simulink model "s_c_nbe_m1k_bu"  (R2016b) (continued)
== system s_c_nbe_m1k_bu == (continued)
  [synced_delay] -> Logical7/2
  [fault_detected] -> Logical7/3
  [sync_timeout] -> Logical14/2
  [auto_rst_enable] -> Logical14/1
  Logical14/1 -> Logical8/2
  Logical7/1 -> Logical8/3
  pipeline23/1(q) -> [outfreq_stopmux]
  ct_debug/6(in_outfreq_stopmux) -> pipeline23/1(d)
  pipeline24/1(q) -> [out_stopmux]
  ct_debug/5(in_out_stopmux) -> pipeline24/1(d)
  pipeline55/1(q) -> [wrrd_stopmux]
  ct_debug/3(in_wrrd_stopmux) -> pipeline55/1(d)
  pipeline56/1(q) -> [in_stopmux]
  ct_debug/1(in_in_stopmux) -> pipeline56/1(d)
  pipeline122/1(q) -> [ct_snap_arm]
  pipeline22/1(q) -> edge_detect7/1
  ct_debug/8(in_snap_trig) -> pipeline22/1(d)
  ct_debug/7(in_snap_arm) -> pipeline122/1(d)
  edge_detect7/1 -> [ct_snap_trig]
  ct_debug/2(in_in_trigmux) -> pipeline150/1(d)
  pipeline150/1(q) -> [in_trigmux]
  ct_debug/4(in_obuf_stopmux) -> pipeline167/1(d)
  pipeline167/1(q) -> [obuf_stopmux]
  ct_debug/9(in_snap_wesel) -> pipeline81/1(d)
  pipeline81/1(q) -> [ct_snap_wesel]
  pipeline157/1(q) -> [ct_snap_trigsel]
  ct_debug/10(in_snap_trigsel) -> pipeline157/1(d)
  pipeline166/1(q) -> [ct_snap_circsel]
  ct_debug/11(in_snap_circsel) -> pipeline166/1(d)
  bus_create2/1(bus_out) -> [tvgtone_level0]
  bus_create3/1(bus_out) -> [tvgtone_level1]
  tvg_tone_levels/2(in_p0i) -> bus_create2/2(in2)
  tvg_tone_levels/3(in_p1r) -> bus_create3/1(in1)
  tvg_tone_levels/4(in_p1i) -> bus_create3/2(in2)
  tvg_tone_levels/1(in_p0r) -> bus_create2/1(in1)
  tvg_tone_targets/1(in_p0) -> Slice18/1
  tvg_tone_targets/2(in_p1) -> Slice19/1
  Constant51/1 -> tvg_tone_targets/1(sim_1)
  Constant52/1 -> tvg_tone_targets/2(sim_2)
  Constant37/1 -> tvg_tone_levels/4(sim_4)
  Constant8/1 -> tvg_tone_levels/1(sim_1)
  Constant27/1 -> tvg_tone_levels/3(sim_3)
  Constant9/1 -> tvg_tone_levels/2(sim_2)
  we_delay49/1(q) -> [ct_tag_insert_sel]
  edge_detect2/1 -> [ct_rst_err_latch]
  we_delay47/1(q) -> edge_detect2/1
  we_delay48/1(q) -> [ct_fake_data_error]
  we_delay13/1(q) -> [ct_tvg_en]
  we_delay50/1(q) -> [ct_tvg_en2]
  Constant12/1 -> ct_control0/5(sim_5)
  Constant21/1 -> ct_control0/4(sim_4)
  Constant20/1 -> ct_control0/3(sim_3)
  Constant22/1 -> ct_control0/2(sim_2)
  Constant15/1 -> ct_control0/1(sim_1)
  ct_control0/1(in_tvg_en) -> we_delay13/1(d)
  ct_control0/2(in_tvg_en2) -> we_delay50/1(d)
  ct_control0/3(in_rst_err_latch) -> we_delay47/1(d)
  ct_control0/4(in_fake_error) -> we_delay48/1(d)
  ct_control0/5(in_tag_insert) -> we_delay49/1(d)
  we_delay126/1(q) -> [obuf_read_gap]
  ct_control0/6(in_obuf_read_gap) -> we_delay126/1(d)
  Constant30/1 -> ct_control0/6(sim_6)
  we_delay41/1(q) -> [ct_num_xeng]
  we_delay112/1(q) -> [ct_num_xeng_recip]  "NUM_X_RECIP"
  ct_control1/2(in_num_x_recip) -> we_delay112/1(d)
  ct_control1/1(in_num_x) -> we_delay41/1(d)
  we_delay114/1(q) -> [ct_x_per_board]
  ct_control1/3(in_x_per_board) -> we_delay114/1(d)
  we_delay113/1(q) -> [ct_chans_per_board]
  ct_control2/1(in_chans_per_x) -> we_delay39/1(d)
  ct_control2/2(in_chans_per_board) -> we_delay113/1(d)
  we_delay115/1(q) -> [ct_x_per_board_recip]
  ct_control1/4(in_x_per_board_recip) -> we_delay115/1(d)
  we_delay38/1(q) -> [ct_num_x_boards]
  ct_control3/1(in_num_x_boards) -> we_delay38/1(d)
  we_delay37/1(q) -> [ct_num_x_boards_recip]
  ct_control3/2(in_num_x_boards_recip) -> we_delay37/1(d)
  ct_control3/3(in_chans_per_x_recip) -> we_delay124/1(d)
  Constant49/1 -> ct_control1/1(sim_1)
  Constant53/1 -> ct_control1/2(sim_2)
  Constant33/1 -> ct_control1/3(sim_3)
  Constant23/1 -> ct_control2/1(sim_1)
  Constant50/1 -> ct_control2/2(sim_2)
  Constant54/1 -> ct_control1/4(sim_4)
  Constant32/1 -> ct_control3/1(sim_1)
  Constant14/1 -> ct_control3/2(sim_2)
  Constant44/1 -> ct_control3/3(sim_3)
  Slice18/1 -> [tvgtone_p0]
  Slice19/1 -> [tvgtone_p1]
  Slice36/1 -> we_delay116/1(d)
  Constant38/1 -> ct_control4/1(sim_1)
  ct_control4/1(in_ct_board_offset) -> Slice36/1
  ct_control5/1(in_ct_freq_gen_offset) -> we_delay117/1(d)
  Constant39/1 -> ct_control5/1(sim_1)
  we_delay116/1(q) -> [ct_board_hmc_offset]
  we_delay117/1(q) -> [ct_freq_gen_offset]
  we_delay124/1(q) -> [ct_chans_per_x_recip]
  we_delay39/1(q) -> [ct_chans_per_x]  "PER_X"
  pipeline196/1(q) -> ddc_dvalid_cnt/1(out_reg)
  pipeline197/1(q) -> ddc_sync_cnt/1(out_reg)
  pipeline198/1(q) -> DDC/8(scale1)
  pipeline199/1(q) -> DDC/5(scale0)
  trig_time_msw/1(in_msw) -> pipeline213/1(d)
  trig_time_lsw/1(in_lsw) -> pipeline214/1(d)
  Concat13/1 -> Relational10/1
  pipeline213/1(q) -> Concat13/1
  pipeline214/1(q) -> Concat13/2
  [time80_48] -> Relational10/2
  pipeline215/1(q) -> bus_expand18/1(bus_in)
  [valid80] -> Slice5/1
  Slice14/1 -> pipeline211/1(d)
  [time80_48] -> Slice14/1
  pipeline211/1(q) -> snap_adc0/13(extra_timestamp)
  [d80_adc0] -> pipeline215/1(d)
  bus_expand18/4(out5) -> snap_adc0/4(in_p0_d3)
  bus_expand18/5(out4) -> snap_adc0/5(in_p0_d4)
  bus_expand18/1(msb_out8) -> Gateway Out3/1, snap_adc0/1(in_p0_d0)
  bus_expand18/2(out7) -> Gateway Out23/1, snap_adc0/2(in_p0_d1)
  bus_expand18/3(out6) -> snap_adc0/3(in_p0_d2)
  bus_expand18/6(out3) -> snap_adc0/6(in_p0_d5)
  bus_expand18/7(out2) -> snap_adc0/7(in_p0_d6)
  bus_expand18/8(lsb_out1) -> snap_adc0/8(in_p0_d7)
  pipeline202/1(q) -> snap_adc0/11(we)
  Relational10/1 -> Logical12/1
  [valid80] -> pipeline162/1(d)
  Logical12/1 -> snap_adc0/12(trig)
  pipeline162/1(q) -> Logical12/2
  [pfbin_sync] -> Delay21/1
  [ddc_sync_in] -> DDC/1(sync)
  [pfbin_d0] -> Delay2/1
  pipeline68/1(q) -> pipeline66/1(d)
  pipeline83/1(q) -> pipeline59/1(d)
  Slice5/1 -> pipeline68/1(d), pipeline202/1(d)
  [sync80] -> pipeline83/1(d)
  [ddc_pol1_in] -> DDC/6(pol1_data)
  Slice17/1 -> local_time_lsw/1(out_timestamp_lsw)
  Slice15/1 -> Convert1/1
  [local_time_capture] -> Delay31/1
  pipeline19/1(q) -> Slice17/1, Slice15/1
  [local_time_src] -> Mux/1
  [time80_48] -> Delay14/1
  [valid80] -> Delay14/2
  Delay14/1 -> Register1/1, AddSub/2
  [pfb_time] -> Delay10/1
  [pfb_dv] -> Delay10/2
  Delay11/1 -> Register3/1, AddSub/1
  [flag_dvalid] -> Delay11/2
  [pack_sync_time] -> Delay11/1
  Convert1/1 -> local_time_msw/1(out_timestamp_msw)
  AddSub/1 -> time_difference/1(out_time_difference), Relational4/1
  Relational4/1 -> Logical/1
  Logical/1 -> [time_diff_error]
  time_check/1(in_max_difference) -> Relational4/2
  [time_diff_check_en] -> Logical/2
  Delay31/1 -> Register3/2, Register2/2, Register4/2, Register1/2
  Delay10/1 -> Register2/1
  [spead_timestamp] -> Register4/1
  Register4/1 -> Mux/2
  Register1/1 -> Mux/3
  Register2/1 -> Mux/4
  Register3/1 -> Mux/5
  Mux/1 -> pipeline19/1(d)
  [cnt_rst] -> Subsystem2/1(rst)
  [synced] -> Delay38/1
  [sync_armed] -> Delay39/1
  [board_in_fault] -> Delay48/1
  [q_sync] -> Subsystem19/5(in3)
  [pfb_sync] -> Subsystem19/3(in1)
  [fdfs_sync] -> Subsystem19/4(in2)
  [sync80] -> Subsystem19/2(in0)
  [cnt_rst] -> Subsystem19/1(rst)
  Subsystem19/2(out1) -> sync_status2/2(out_sync_pfb_cnt)
  Subsystem19/1(out0) -> sync_status2/1(out_sync80_cnt)
  Subsystem19/3(out2) -> sync_status2/3(out_sync_fdfs_cnt)
  [pack_sync] -> Subsystem12/4(in2)
  [flag_sync] -> Subsystem12/3(in1)
  [ct_sync] -> Subsystem12/2(in0)
  [cnt_rst] -> Subsystem12/1(rst)
  [synced_delay] -> Subsystem2/2(armed)
  [sync_timeout] -> Subsystem2/3(in1)
  [time_diff_error] -> Subsystem2/4(in2)
  [up_timestep_error] -> Subsystem2/5(in3)
  [cd_error] -> Subsystem2/6(in4)
  [ct_error] -> Subsystem2/7(in5)
  Delay48/1 -> sync_status0/2(out_board_in_fault)
  Delay38/1 -> sync_status0/3(out_synced)
  Delay39/1 -> sync_status0/4(out_sync_armed)
  Subsystem12/1(out0) -> sync_status0/5(out_sync_ct)
  Subsystem12/2(out1) -> sync_status0/6(out_sync_flag)
  Subsystem12/3(out2) -> sync_status0/7(out_sync_pack)
  Subsystem2/1(Out1) -> sync_status1/1(out_timeout_resync_cnt)
  Subsystem2/2(Out2) -> sync_status1/2(out_time_diff_resync_cnt)
  Subsystem2/3(Out3) -> sync_status1/3(out_timestep_resync_cnt)
  [synced_delay] -> Delay58/1
  Delay58/1 -> sync_status0/1(out_synced_delay)
  Subsystem19/4(out3) -> sync_status2/4(out_sync_quant_cnt)
  Subsystem2/4(Out4) -> sync_status1/4(out_cd_resync_cnt)
  Subsystem2/5(Out5) -> sync_status1/5(out_ct_resync_cnt)
  [clr_status] -> Subsystem3/1(rst)
  Slice6/1 -> Subsystem3/2(in0)
  [pfb_of] -> Slice6/1, Slice20/1
  Slice20/1 -> Subsystem3/3(in1)
  [pfb_sync] -> Subsystem3/4(in2)
  Subsystem3/3(out2) -> pipeline193/1(d)
  Subsystem3/1(out0) -> pipeline195/1(d)
  Subsystem3/2(out1) -> pipeline194/1(d)
  Constant17/1 -> [pfb_of]
  Counter7/1 -> pipeline191/1(d)
  [pfb_sync] -> Counter7/2
  [cnt_rst] -> Counter7/1
  [pfb_dv] -> Counter8/2
  [cnt_rst] -> Counter8/1
  Counter8/1 -> pipeline192/1(d)
  pipeline191/1(q) -> nb_sync_cnt/1(out_reg)
  pipeline192/1(q) -> nb_dvalid_cnt/1(out_reg)
  pipeline193/1(q) -> pfb_status/3(out_sync_cnt)
  pipeline194/1(q) -> pfb_status/2(out_pol1_or_err_cnt)
  pipeline195/1(q) -> pfb_status/1(out_pol0_or_err_cnt)
  Delay72/1 -> [flag_sync]
  Delay75/1 -> [flag_misc]
  Delay73/1 -> [flag_dvalid]
  Delay74/1 -> [flag_time]
  Delay43/1 -> time_gen1/4(rd_sync_in)
  Delay59/1 -> time_gen1/6(rd_misc_in)
  Delay/1 -> Delay43/1  "gapsync"
  Delay3/1 -> Delay47/1  "gapdata"
  Mux1/1 -> Delay59/1  "gapvalid"
  [time80] -> Slice2/1
  Delay47/1 -> time_gen1/5(rd_en)
  time_gen1/1(rd_sync_out) -> Delay72/1
  time_gen1/4(rd_misc_out) -> Delay75/1
  time_gen1/2(rd_dvalid) -> Delay73/1
  time_gen1/3(rd_val_out) -> Delay74/1
  Slice2/1 -> time_gen1/3(wr_val_in)
  [ct_tvg_en2] -> Mux1/1
  Delay17/1 -> Mux1/2
  Delay18/1 -> tvg/1(sync), Delay/1
  Delay16/1 -> tvg/2(dv), Delay3/1
  tvg/1(data256) -> Mux1/3
  [flag_dvalid] -> Subsystem13/1(In1)
  Subsystem13/1(Out1) -> pipeline186/1(d)
  [s256_v] -> Subsystem11/1(In1)
  Subsystem11/1(Out1) -> pipeline187/1(d)
  pipeline38/1(q) -> Convert3/1
  pipeline46/1(q) -> [ip_per_x]
  pipeline47/1(q) -> [num_x]
  x_setup/1(in_f_per_x) -> pipeline38/1(d)
  x_setup/2(in_ip_per_x) -> pipeline46/1(d)
  x_setup/3(in_num_x) -> pipeline47/1(d)
  pipeline52/1(q) -> [pack_snap_arm]
  x_setup/4(in_snap_arm) -> pipeline52/1(d)
  pipeline48/1(q) -> [pktstart_sel]
  x_setup/5(in_pktstart_mux) -> pipeline48/1(d)
  [s256_v] -> pack_block_check/1(dv)
  [cnt_rst] -> Subsystem14/1(rst)
  Subsystem14/1(out0) -> pipeline188/1(d)
  pack_block_check/1(error) -> [pack_dv_err], Subsystem14/2(in0)
  pipeline49/1(q) -> [pack_mux]
  x_setup/6(in_pack_mux) -> pipeline49/1(d)
  Convert3/1 -> [f_per_x]
  [ct_d256] -> Delay17/1
  [ct_dv] -> Delay16/1
  [ct_sync] -> Delay18/1
  x_setup/7(in_pktoffset_sel) -> pipeline54/1(d)
  pipeline54/1(q) -> [pktoffset_sel]
  iptx_base/1(in_reg) -> pipeline53/1(d)
  pipeline53/1(q) -> [tx_ip_base]
  tx_metadata/1(in_porttx) -> pipeline50/1(d)
  tx_metadata/2(in_board_id) -> pipeline51/1(d)
  pipeline50/1(q) -> [tx_port]
  pipeline51/1(q) -> [tx_board_id]
  Delay64/1 -> Delay68/1
  Delay4/1 -> Delay5/1
  Delay5/1 -> Delay60/1
  Delay60/1 -> Delay64/1
  Delay68/1 -> [flag_pktstart]
  Delay1/1 -> Delay6/1
  Delay6/1 -> Delay61/1
  Delay65/1 -> Delay69/1
  Delay69/1 -> [flag_pktfreq]
  Delay61/1 -> Delay65/1
  Delay15/1 -> Delay7/1
  Delay7/1 -> Delay62/1
  Delay62/1 -> Delay66/1
  Delay66/1 -> Delay70/1
  Delay70/1 -> [flag_pktxindex]
  Delay19/1 -> Delay20/1
  Delay20/1 -> Delay63/1
  Delay63/1 -> Delay67/1
  Delay67/1 -> Delay71/1
  Delay71/1 -> [flag_pktxoffset]
  [ct_pkt_x_index] -> Delay15/1
  [ct_pkt_x_offset] -> Delay19/1
  [ct_pktfreq] -> Delay1/1
  [ct_pktstart] -> Delay4/1
  [flag_sync] -> packetiser/1(sync_in)
  [flag_dvalid] -> packetiser/2(en)
  [flag_pktstart] -> packetiser/5(pkt_start)
  [flag_pktxoffset] -> packetiser/7(pkt_x_offset)
  [tx_ip_base] -> packetiser/9(base_ip)
  [tx_port] -> packetiser/10(base_port)
  Constant3/1 -> concat1/2
  [flag_time] -> concat1/1
  [flag_misc] -> packetiser/3(din)
  concat1/1 -> [pack_sync_time], packetiser/4(timestamp)
  Delay82/1 -> [s256_d]
  Delay83/1 -> [s256_v]
  Delay84/1 -> [s256_eof]
  Delay85/1 -> [s256_of]
  Delay86/1 -> [s256_ip]
  Delay87/1 -> [s256_port]
  Delay88/1 -> [pack_sync]
  packetiser/1(dout) -> Delay82/1
  packetiser/2(dvalid) -> Delay83/1
  packetiser/3(eof) -> Delay84/1
  packetiser/4(overflow) -> Delay85/1
  packetiser/5(destination_ip) -> Delay86/1
  packetiser/6(destination_port) -> Delay87/1
  packetiser/7(sync) -> Delay88/1
  [flag_pktfreq] -> Delay77/1
  Delay89/1 -> [s256_pktfreq]
  Delay77/1 -> Delay89/1
  [flag_pktxindex] -> Delay78/1
  Delay78/1 -> Delay90/1
  Delay90/1 -> [s256_pktxindex]
  [flag_pktxoffset] -> Delay79/1
  Delay79/1 -> Delay91/1
  Delay91/1 -> [s256_pktxoffset]
  [tx_board_id] -> packetiser/8(board_id)
  [flag_pktstart] -> Delay80/1
  Delay80/1 -> Delay92/1
  Delay92/1 -> [s256_pktstart]
  [sync80] -> time_gen1/1(wr_sync_in)
  [valid80] -> time_gen1/2(wr_en)
  [time80_48] -> Subsystem/1(time)
  [sync80] -> Subsystem/2(sync)
  [valid80] -> Subsystem/3(dv)
  Subsystem/1(msw) -> pipeline184/1(d)
  Subsystem/2(ls32) -> pipeline185/1(d)
  [flag_pktxindex] -> packetiser/6(pkt_x_index)
  [flag_time] -> Delay81/1
  Delay81/1 -> Delay93/1
  Delay93/1 -> [s256_time]
  time_gen1/5(epoch) -> Delay76/1
  Slice4/1 -> pipeline182/1(d)
  Slice11/1 -> pipeline183/1(d)
  Delay76/1 -> Concat10/1
  Constant16/1 -> Concat10/2
  Concat10/1 -> Slice4/1, Slice11/1, [flag_epoch]
  [flag_pktfreq] -> Subsystem27/3(value)
  [cnt_rst] -> Subsystem27/1(rst)
  [flag_dvalid] -> Subsystem27/2(en)
  Subsystem27/1(current) -> pipeline170/1(d)
  Subsystem27/3(min) -> pipeline172/1(d)
  Subsystem27/2(max) -> pipeline171/1(d)
  [flag_pktxindex] -> Subsystem28/3(value)
  [cnt_rst] -> Subsystem28/1(rst)
  [flag_dvalid] -> Subsystem28/2(en)
  Subsystem28/1(current) -> pipeline173/1(d)
  Subsystem28/3(min) -> pipeline175/1(d)
  Subsystem28/2(max) -> pipeline174/1(d)
  [flag_pktxoffset] -> Subsystem29/3(value)
  [cnt_rst] -> Subsystem29/1(rst)
  [flag_dvalid] -> Subsystem29/2(en)
  Subsystem29/1(current) -> pipeline176/1(d)
  Subsystem29/3(min) -> pipeline178/1(d)
  Subsystem29/2(max) -> pipeline177/1(d)
  [flag_time] -> Subsystem30/3(value)
  [cnt_rst] -> Subsystem30/1(rst)
  [flag_pktstart] -> Subsystem30/2(en)
  Subsystem30/1(current) -> pipeline179/1(d)
  Subsystem30/3(min) -> pipeline181/1(d)
  Subsystem30/2(max) -> pipeline180/1(d)
  pipeline170/1(q) -> flag_pktfreq_stats/1(out_current)
  pipeline171/1(q) -> flag_pktfreq_stats/2(out_max)
  pipeline172/1(q) -> flag_pktfreq_stats/3(out_min)
  pipeline175/1(q) -> flag_pktxindex_stats/3(out_min)
  pipeline174/1(q) -> flag_pktxindex_stats/2(out_max)
  pipeline173/1(q) -> flag_pktxindex_stats/1(out_current)
  pipeline176/1(q) -> flag_pktxoffset_stats/1(out_current)
  pipeline177/1(q) -> flag_pktxoffset_stats/2(out_max)
  pipeline178/1(q) -> flag_pktxoffset_stats/3(out_min)
  pipeline181/1(q) -> flag_time_stats/3(out_min)
  pipeline180/1(q) -> flag_time_stats/2(out_max)
  pipeline179/1(q) -> flag_time_stats/1(out_current)
  pipeline182/1(q) -> flag_epoch_lsw/1(out_reg)
  pipeline183/1(q) -> flag_epoch_msw/1(out_reg)
  pipeline184/1(q) -> sync_time_msw/1(out_reg)
  pipeline185/1(q) -> sync_time_lsw/1(out_reg)
  pipeline186/1(q) -> pack_in_dv_rate/1(out_dv_rate)
  pipeline187/1(q) -> pack_out_dv_rate/1(out_dv_rate)
  pipeline188/1(q) -> pack_dv_err/1(out_dvblock_err_cnt)
  [pfbin_sync] -> Logical9/2
  [sys_rst] -> Logical9/1
  Logical9/1 -> pipeline147/1(d)
  [osc_cos_ddc0] -> DDC/7(osc1)
  Mux2/1 -> DDC/4(osc0)
  [osc_cos_ddc0] -> Mux2/2
  [ddc_pol0_in] -> Mux2/3
  Constant11/1 -> osc_sel/1(sim_1)
  osc_sel/1(in_sel) -> Mux2/1
  [pfbin_dv] -> Delay9/1
  Delay2/1 -> [ddc_pol0_in]
  Delay9/1 -> [ddc_dv_in]
  Delay21/1 -> [ddc_sync_in]
  [ddc_pol0] -> nb_pfb/2(pol0)
  [ddc_pol1] -> nb_pfb/3(pol1)
  nb_pfb/2(pol0_out) -> [pfb_d144_0]
  nb_pfb/3(pol1_out) -> [pfb_d144_1]
  [ddc_sync] -> nb_pfb/1(sync)
  nb_pfb/1(sync_out) -> [pfb_sync]
  [ddc_dvalid] -> nb_pfb/4(dvalid)
  nb_pfb/4(dv_out) -> [pfb_dv]
  pipeline204/1(q) -> snap_fft/5(we)
  pipeline203/1(q) -> snap_fft/1(in_real)
  pipeline206/1(q) -> snap_fft/2(in_imag)
  pipeline20/1(q) -> snap_fft/3(in_sync)
  pipeline216/1(q) -> snap_fft/4(in_dv)
  bus_expand1/2(lsb_out1) -> pipeline206/1(d)
  bus_expand1/1(msb_out2) -> pipeline203/1(d)
  bus_expand2/1(msb_out8) -> pipeline142/1(d)
  bus_expand2/2(out7) -> pipeline144/1(d)
  bus_expand2/3(out6) -> pipeline145/1(d)
  bus_expand2/4(out5) -> pipeline146/1(d)
  bus_expand2/5(out4) -> pipeline151/1(d)
  bus_expand2/6(out3) -> pipeline152/1(d)
  pipeline142/1(q) -> snap_nb/1(in_real0)
  pipeline144/1(q) -> snap_nb/2(in_imag0)
  pipeline146/1(q) -> snap_nb/4(in_imag1)
  pipeline151/1(q) -> snap_nb/5(in_real2)
  pipeline152/1(q) -> snap_nb/6(in_imag2)
  pipeline4/1(q) -> snap_nb/7(we)
  generate_pkt_sync1/2(out_valid) -> pipeline34/1(d)
  pipeline154/1(q) -> snap_nb1/1(in_real3)
  pipeline217/1(q) -> snap_nb1/2(in_imag3)
  bus_expand2/7(out2) -> pipeline154/1(d)
  bus_expand2/8(lsb_out1) -> pipeline217/1(d)
  pipeline5/1(q) -> snap_nb1/5(we)
  pipeline229/1(q) -> snap_nb1/4(in_dv)
  pipeline230/1(q) -> snap_nb1/3(in_sync)
  generate_pkt_sync1/4(packet_sync) -> pipeline36/1(d)
  [ddc_dv_in] -> DDC/2(dvalid)
  pipeline145/1(q) -> snap_nb/3(in_real1)
  [ddc_pol0_in] -> DDC/3(pol0_data)
  [pfbin_d1] -> Delay22/1
  [pfbin_dv] -> cwg1/6(en)
  Delay22/1 -> [ddc_pol1_in]
  pipeline106/1(q) -> [osc_temp]
  cwg2/1(bus_out) -> pipeline106/1(d)
  cwg2/3(rpt_rst) -> Terminator15/1
  cwg2/2(dvalid) -> Terminator8/1
  Constant56/1 -> freq_cwg_osc1/1(sim_1)
  Constant58/1 -> scale_cwg_osc1/1(sim_1)
  freq_cwg_osc1/1(in_frequency) -> pipeline121/1(d)
  scale_cwg_osc1/1(in_scale) -> pipeline99/1(d)
  pipeline119/1(q) -> cwg2/3(rst)
  rpt_en_cwg_osc1/1(in_en) -> pipeline123/1(d)
  rpt_length_cwg_osc1/1(in_repeat_length) -> pipeline105/1(d)
  Constant59/1 -> rpt_en_cwg_osc1/1(sim_1)
  Constant60/1 -> rpt_length_cwg_osc1/1(sim_1)
  pipeline99/1(q) -> cwg2/2(scale)
  pipeline121/1(q) -> cwg2/1(freq)
  pipeline123/1(q) -> cwg2/4(repeat_en)
  pipeline105/1(q) -> cwg2/5(repeat_length)
  [pfbin_sync] -> Logical10/2
  [sys_rst] -> Logical10/1
  Logical10/1 -> pipeline119/1(d)
  Gateway Out3/1 -> To Workspace4/1
  Gateway Out23/1 -> To Workspace23/1
  bus_expand6/4(out5) -> pipeline139/1(d)
  bus_expand6/5(out4) -> pipeline133/1(d)
  bus_expand6/2(out7) -> pipeline141/1(d)
  bus_expand6/3(out6) -> pipeline140/1(d)
  bus_expand6/6(out3) -> pipeline130/1(d)
  bus_expand6/7(out2) -> pipeline129/1(d)
  bus_expand6/8(lsb_out1) -> pipeline126/1(d)
  bus_expand6/1(msb_out8) -> pipeline149/1(d)
  pipeline153/1(q) -> snap_hmc_in/12(we)
  pipeline124/1(q) -> snap_hmc_in/10(in_dv)
  pipeline125/1(q) -> snap_hmc_in/9(in_sync)
  pipeline126/1(q) -> snap_hmc_in/8(in_p0_d7)
  pipeline129/1(q) -> snap_hmc_in/7(in_p0_d6)
  pipeline130/1(q) -> snap_hmc_in/6(in_p0_d5)
  pipeline133/1(q) -> snap_hmc_in/5(in_p0_d4)
  pipeline139/1(q) -> snap_hmc_in/4(in_p0_d3)
  pipeline140/1(q) -> snap_hmc_in/3(in_p0_d2)
  pipeline141/1(q) -> snap_hmc_in/2(in_p0_d1)
  pipeline149/1(q) -> snap_hmc_in/1(in_p0_d0)
  From131/1 -> pipeline125/1(d), snap_hmc_in/13(trig)
  From129/1 -> pipeline124/1(d), pipeline153/1(d)
  pipeline161/1(q) -> snap_hmc_in/11(in_en)
  From189/1 -> pipeline161/1(d)
  From186/1 -> bus_expand6/1(bus_in)
  Mux7/1 -> Delay36/1, cwg2/6(en)
  Delay36/1 -> Gateway Out28/1, [osc_temp_dv]
  Counter10/1 -> Relational5/1, Relational2/1, Gateway Out26/1
  Constant62/1 -> osc_temp_dv_sel/1(sim_1)
  osc_temp_dv_sel/1(in_sel) -> Mux7/1
  Constant/1 -> Mux7/2
  Relational2/1 -> Mux7/3, Gateway Out29/1
  Gateway Out28/1 -> To Workspace28/1
  Register5/1 -> edge_detect10/1(in)
  [osc_temp_dv] -> Register5/3, Register5/1
  [sys_rst] -> Register5/2
  Mux8/1 -> [sync80]
  edge_detect10/1(out) -> Mux8/3
  Mux9/1 -> [valid80]
  [osc_temp_dv] -> Delay24/1
  Mux10/1 -> [d80_adc0]
  [osc_temp] -> Delay25/1
  Constant61/1 -> dbg_sel/1(sim_1)
  dbg_sel/1(in_sel) -> Mux8/1, Mux9/1, Mux10/1
  From77/1 -> bus_expand1/1(bus_in)
  From46/1 -> pipeline204/1(d), pipeline216/1(d)
  From45/1 -> snap_fft/6(trig), pipeline20/1(d)
  Counter11/1 -> pipeline233/1(d)
  From98/1 -> Counter11/2
  From117/1 -> Counter11/1
  From101/1 -> Counter13/2
  From118/1 -> Counter13/1
  bus_expand9/4(out5) -> pipeline241/1(d)
  bus_expand9/5(out4) -> pipeline240/1(d)
  bus_expand9/2(out7) -> pipeline243/1(d)
  bus_expand9/3(out6) -> pipeline242/1(d)
  bus_expand9/6(out3) -> pipeline239/1(d)
  bus_expand9/7(out2) -> pipeline238/1(d)
  bus_expand9/8(lsb_out1) -> pipeline32/1(d)
  bus_expand9/1(msb_out8) -> pipeline244/1(d)
  pipeline245/1(q) -> snap_hmc_out/12(we)
  pipeline21/1(q) -> snap_hmc_out/10(in_dv)
  pipeline26/1(q) -> snap_hmc_out/9(in_sync)
  pipeline32/1(q) -> snap_hmc_out/8(in_p0_d7)
  pipeline238/1(q) -> snap_hmc_out/7(in_p0_d6)
  pipeline239/1(q) -> snap_hmc_out/6(in_p0_d5)
  pipeline240/1(q) -> snap_hmc_out/5(in_p0_d4)
  pipeline241/1(q) -> snap_hmc_out/4(in_p0_d3)
  pipeline242/1(q) -> snap_hmc_out/3(in_p0_d2)
  pipeline243/1(q) -> snap_hmc_out/2(in_p0_d1)
  pipeline244/1(q) -> snap_hmc_out/1(in_p0_d0)
  From241/1 -> pipeline246/1(d)
  pipeline246/1(q) -> snap_hmc_out/11(in_err)
  From235/1 -> bus_expand9/1(bus_in)
  From232/1 -> pipeline245/1(d), pipeline21/1(d)
  From239/1 -> snap_hmc_out/13(trig), pipeline26/1(d)
  Counter15/1 -> pipeline236/1(d)
  [ddc_sync_in] -> Counter15/2
  [sys_rst] -> Counter15/1
  tl_fd0/2(enable) -> phase_compensation0/5(load_value)
  tl_fd1/1(value) -> phase_compensation1/4(delay_value)
  tl_fd1/2(enable) -> phase_compensation1/5(load_value)
  tl_fd0/1(value) -> phase_compensation0/4(delay_value)
  [clr_status] -> tl_fd1/1(rst), tl_fd0/1(rst)
  phase_compensation0/1(sync_out) -> [fdfs_sync]
  phase_compensation1/1(sync_out) -> Terminator24/1
  phase_compensation0/2(dout) -> [fdfs_d144_0]
  phase_compensation1/2(dout) -> [fdfs_d144_1]
  phase_compensation0/3(dvalid) -> [fdfs_dv]
  phase_compensation1/3(dvalid) -> Terminator25/1
  [ld_val_fd0] -> tl_fd0/3(load_value)
  [ld_val_fd1] -> tl_fd1/3(load_value)
  bus_expand16/1(msb_out2) -> tl_fd0/5(din)
  tl_fd0/5(dvalid) -> phase_compensation0/3(en)
  tl_fd0/3(sync_out) -> phase_compensation0/1(sync_in)
  tl_fd0/4(dout) -> phase_compensation0/2(din)
  tl_fd1/4(dout) -> phase_compensation1/2(din)
  bus_expand16/2(lsb_out1) -> tl_fd1/5(din)
  Constant66/1 -> Concat2/2
  Concat2/1 -> tl_fd1/2(current_time), tl_fd0/2(current_time), [pfb_time]
  Counter2/1 -> pipeline41/1(d)
  Constant65/1 -> eq1/2(data_in)
  Constant24/1 -> eq1/3(we)
  Delay53/1 -> quant1/1(sync_in)
  Delay37/1 -> quant1/2(din)
  eq1/1(data_out) -> quant1/4(coeffs)
  Delay50/1 -> quant1/3(en)
  Counter4/1 -> pipeline29/1(d)
  Constant63/1 -> eq0/2(data_in)
  Constant64/1 -> eq0/3(we)
  Delay51/1 -> quant0/1(sync_in)
  Delay35/1 -> quant0/2(din)
  Delay52/1 -> quant0/3(en)
  quant0/1(sync_out) -> [q_sync]
  quant0/2(dout) -> [q_d64_0]
  quant0/3(dvalid) -> [q_dv]
  quant0/4(of) -> [q_of0]
  quant1/2(dout) -> [q_d64_1]
  quant1/1(sync_out) -> Terminator19/1
  quant1/3(dvalid) -> Terminator21/1
  quant1/4(of) -> [q_of1]
  pipeline29/1(q) -> eq0/1(addr)
  pipeline41/1(q) -> eq1/1(addr)
  [fdfs_sync] -> Delay51/1, pipeline45/1(d)
  [fdfs_d144_0] -> Delay35/1
  [fdfs_dv] -> Delay52/1, pipeline42/1(d)
  pipeline42/1(q) -> Counter4/2
  pipeline45/1(q) -> Counter4/1
  pipeline189/1(q) -> Counter2/2
  [fdfs_sync] -> Delay53/1, pipeline200/1(d)
  [fdfs_d144_1] -> Delay37/1
  [fdfs_dv] -> Delay50/1, pipeline189/1(d)
  pipeline200/1(q) -> Counter2/1
  [clr_status] -> Subsystem15/1(rst)
  tl_fd0/7(misco) -> Terminator3/1
  tl_fd0/6(time) -> Terminator4/1
  tl_fd1/6(time) -> Terminator17/1
  tl_fd1/3(sync_out) -> phase_compensation1/1(sync_in)
  tl_fd1/5(dvalid) -> phase_compensation1/3(en)
  tl_fd1/7(misco) -> Terminator20/1
  Constant26/1 -> tl_fd0/7(misci)
  Constant29/1 -> tl_fd1/7(misci)
  time_gen/1(rd_sync_out) -> tl_fd1/4(sync_in), tl_fd0/4(sync_in)
  time_gen/2(rd_dvalid) -> tl_fd1/6(en), tl_fd0/6(en)
  time_gen/3(rd_val_out) -> Concat2/1
  time_gen/4(rd_misc_out) -> bus_expand16/1(bus_in)
  [sync80] -> pipeline207/1(d)
  [valid80] -> pipeline208/1(d)
  [pfb_sync] -> pipeline210/1(d)
  [pfb_dv] -> pipeline212/1(d)
  [pfb_d144_1] -> pipeline231/1(d)
  [pfb_d144_0] -> pipeline228/1(d)
  Concat1/1 -> time_gen/6(rd_misc_in)
  [time80] -> pipeline209/1(d)
  Subsystem15/1(out0) -> quant_status/1(out_pol0_or_cnt)
  Relational/1 -> Subsystem15/2(in0)
  Constant4/1 -> Relational/2
  [q_of0] -> Relational/1
  Relational3/1 -> Subsystem15/3(in1)
  [q_of1] -> Relational3/1
  Constant25/1 -> Relational3/2
  Subsystem15/2(out1) -> quant_status/2(out_pol1_or_cnt)
  [q_sync] -> Gateway Out36/1, generate_pkt_sync0/1(sync)
  Concat/1 -> generate_pkt_sync0/3(data)
  generate_pkt_sync0/1(out_sync) -> Terminator18/1
  [q_d64_0] -> Concat/1
  [q_d64_1] -> Concat/2
  bus_expand14/1(msb_out2) -> bus_expand12/1(bus_in)
  [q_dv] -> Gateway Out37/1, generate_pkt_sync0/2(valid)
  generate_pkt_sync0/3(out_data) -> pipeline190/1(d)
  generate_pkt_sync0/2(out_valid) -> pipeline201/1(d)
  generate_pkt_sync0/4(packet_sync) -> pipeline205/1(d)
  pipeline190/1(q) -> bus_expand14/1(bus_in)
  pipeline201/1(q) -> Gateway Out31/1, snap_quant0/9(we)
  pipeline205/1(q) -> Gateway Out27/1, snap_quant0/10(trig)
  bus_expand12/1(msb_out8) -> Gateway Out32/1, snap_quant0/1(in_real0)
  bus_expand12/2(out7) -> Gateway Out33/1, snap_quant0/2(in_imag0)
  bus_expand12/3(out6) -> snap_quant0/3(in_real1)
  bus_expand12/4(out5) -> snap_quant0/4(in_imag1)
  bus_expand12/5(out4) -> snap_quant0/5(in_real2)
  bus_expand12/6(out3) -> snap_quant0/6(in_imag2)
  bus_expand12/7(out2) -> Gateway Out34/1, snap_quant0/7(in_real3)
  bus_expand12/8(lsb_out1) -> Gateway Out35/1, snap_quant0/8(in_imag3)
  bus_expand13/1(msb_out8) -> snap_quant1/1(in_real0)
  bus_expand13/2(out7) -> snap_quant1/2(in_imag0)
  bus_expand13/3(out6) -> snap_quant1/3(in_real1)
  bus_expand13/4(out5) -> snap_quant1/4(in_imag1)
  bus_expand13/5(out4) -> snap_quant1/5(in_real2)
  bus_expand13/6(out3) -> snap_quant1/6(in_imag2)
  bus_expand13/7(out2) -> snap_quant1/7(in_real3)
  bus_expand13/8(lsb_out1) -> snap_quant1/8(in_imag3)
  [tl_cd0_arm] -> tl_fd0/8(arm)
  [tl_cd0_load_immediate] -> tl_fd0/9(load_immediate)
  [tl_cd0_load_time] -> tl_fd0/10(load_time)
  [tl_cd1_arm] -> tl_fd1/8(arm)
  [tl_cd1_load_immediate] -> tl_fd1/9(load_immediate)
  [tl_cd1_load_time] -> tl_fd1/10(load_time)
  pipeline207/1(q) -> time_gen/1(wr_sync_in)
  pipeline208/1(q) -> time_gen/2(wr_en)
  pipeline209/1(q) -> time_gen/3(wr_val_in)
  pipeline210/1(q) -> time_gen/4(rd_sync_in)
  pipeline212/1(q) -> time_gen/5(rd_en)
  pipeline228/1(q) -> Concat1/1
  pipeline231/1(q) -> Concat1/2
  Counter13/1 -> pipeline232/1(d)
  pipeline233/1(q) -> hmc_out_sync_cnt/1(out_reg)
  pipeline236/1(q) -> ddc_in_sync_cnt/1(out_reg)
  pipeline232/1(q) -> sync80_cnt/1(out_reg)
  Counter14/1 -> pipeline237/1(d)
  [q_sync] -> Counter14/2
  [sys_rst] -> Counter14/1
  pipeline237/1(q) -> quant_sync_cnt/1(out_reg)
  From_pfb_dv1/1 -> bus_expand13/1(bus_in)
  From_pfb_dv2/1 -> snap_quant1/9(we)
  From_pfb_sync2/1 -> snap_quant1/10(trig)
  Concat4/1 -> [ld_val_fd0]
  Concat9/1 -> [ld_val_fd1]
  fd0_phase/1(in_initial) -> Reinterpret10/1
  fd0_delta_delay/1(in_delta) -> Reinterpret9/1
  fd0_phase/2(in_delta) -> Reinterpret11/1
  Reinterpret16/1 -> Concat4/1
  Reinterpret9/1 -> Concat4/2
  Reinterpret10/1 -> Concat4/3
  Reinterpret11/1 -> Concat4/4
  fd1_phase/1(in_initial) -> Reinterpret13/1
  fd1_delta_delay/1(in_delta) -> Reinterpret12/1
  fd1_phase/2(in_delta) -> Reinterpret14/1
  Reinterpret12/1 -> Concat9/2
  Reinterpret13/1 -> Concat9/3
  Reinterpret14/1 -> Concat9/4
  Reinterpret15/1 -> Concat9/1
  fd0_delay/1(in_initial) -> Reinterpret16/1
  fd1_delay/1(in_initial) -> Reinterpret15/1
  [ct_dv] -> Subsystem21/1(In1)
  Subsystem21/1(Out1) -> ct_out_dv_rate1/1(out_reg)
  [ct_pktstart] -> we_delay3/1(d)
  [ct_dv] -> we_delay4/1(d)
  we_delay3/1(q) -> pktlen_check1/1(pkt_start)
  we_delay4/1(q) -> dv_block_check1/1(dv), pktlen_check1/2(dv)
  pktlen_check1/1(error) -> we_delay6/1(d)
  dv_block_check1/1(error) -> we_delay5/1(d)
  [cnt_rst] -> Subsystem20/1(rst)
  we_delay6/1(q) -> [ct_pktlen_err], Subsystem20/2(in0)
  we_delay5/1(q) -> [ct_dvblk_err], Subsystem20/3(in1)
  Subsystem20/1(out0) -> ct_dv_err1/1(out_pktlen_err_cnt)
  Subsystem20/2(out1) -> ct_dv_err1/2(out_dvblock_err_cnt)
  [q_dv] -> Subsystem18/1(In1)
  Subsystem18/1(Out1) -> ct_in_dv_rate1/1(out_reg)
  [q_sync] -> hmc_ct/1(in_sync)
  [q_dv] -> hmc_ct/2(in_dv)
  [q_d64_0] -> hmc_ct/3(in_d0)
  [q_d64_1] -> hmc_ct/4(in_d1)
  hmc_ct/1(out_sync) -> [ct_sync]
  hmc_ct/2(out_dv) -> [ct_dv]
  hmc_ct/3(out_d256) -> [ct_d256]
  hmc_ct/4(pkt_start) -> [ct_pktstart]
  hmc_ct/5(freq_id) -> [ct_pktfreq]
  hmc_ct/6(x_index) -> [ct_pkt_x_index]
  hmc_ct/7(x_pkt_index) -> [ct_pkt_x_offset]
  hmc_ct/8(error0) -> [ct_error]
  [cnt_rst] -> Subsystem16/1(rst)
  [q_sync] -> Subsystem16/2(in0)
  Constant67/1 -> Subsystem16/3(in1)
  Constant68/1 -> Subsystem16/4(in2)
  [ct_sync] -> Subsystem16/5(in3)
  Subsystem16/1(out0) -> ct_sync_cnt1/1(out_sync_in)
  Subsystem16/2(out1) -> ct_sync_cnt1/2(out_rd_addr_arm_cnt)
  Subsystem16/3(out2) -> ct_sync_cnt1/3(out_rd_sync_cnt)
  Subsystem16/4(out3) -> ct_sync_cnt1/4(out_sync_out)
  munge_sel/1(in_sel) -> pipeline25/1(d)
  pipeline25/1(q) -> [munge_sel]
  Convert2/1 -> [cd_bypass]
  Delay24/1 -> Mux9/3
  Delay25/1 -> Mux10/3
  [pfb_sync] -> generate_pkt_sync1/1(sync)
  [pfb_dv] -> generate_pkt_sync1/2(valid)
  [pfb_d144_0] -> generate_pkt_sync1/3(data)
  generate_pkt_sync1/3(out_data) -> pipeline35/1(d)
  generate_pkt_sync1/1(out_sync) -> Terminator2/1
  pipeline16/1(q) -> snap_fft1/7(we)
  pipeline11/1(q) -> snap_fft1/1(in_real)
  pipeline27/1(q) -> snap_fft1/2(in_imag)
  pipeline10/1(q) -> snap_fft1/5(in_sync)
  pipeline33/1(q) -> snap_fft1/6(in_dv)
  bus_expand3/2(lsb_out1) -> pipeline27/1(d)
  bus_expand3/1(msb_out2) -> pipeline11/1(d)
  generate_pkt_sync2/3(out_data) -> pipeline40/1(d)
  generate_pkt_sync2/2(out_valid) -> pipeline37/1(d)
  generate_pkt_sync2/4(packet_sync) -> pipeline44/1(d)
  [data_pol0] -> generate_pkt_sync2/3(data)
  [fft_sync] -> generate_pkt_sync2/1(sync)
  [fft_dvalid] -> generate_pkt_sync2/2(valid)
  generate_pkt_sync2/1(out_sync) -> Terminator5/1
  pipeline34/1(q) -> pipeline4/1(d), Gateway Out38/1, pipeline5/1(d), pipeline229/1(d)
  pipeline35/1(q) -> bus_expand2/1(bus_in)
  pipeline36/1(q) -> pipeline230/1(d), Gateway Out39/1, snap_nb1/6(trig), snap_nb/8(trig)
  pipeline37/1(q) -> pipeline33/1(d), pipeline16/1(d)
  pipeline40/1(q) -> bus_expand3/1(bus_in)
  pipeline44/1(q) -> pipeline10/1(d), snap_fft1/8(trig)
  [sync80] -> [pfbin_sync]
  [valid80] -> [pfbin_dv]
  [d80_adc0] -> [pfbin_d0]
  [d80_adc1] -> [pfbin_d1]
  Constant5/1 -> [cd_error]
  Constant10/1 -> munge_sel/1(sim_1)
  pipeline59/1(q) -> snap_adc0/9(in_sync)
  pipeline66/1(q) -> snap_adc0/10(in_dv)
  pipeline8/1(q) -> [unpack_dv]
  pipeline17/1(q) -> [unpack_timestamp]
  pipeline14/1(q) -> [tvg_adc0_en]
  impulse_gen0/1(sync_out) -> Delay23/1, Mux3/2
  Mux3/1 -> Mux8/2
  Delay23/1 -> Mux3/3
  Mux4/1 -> Mux9/2
  impulse_gen0/3(dvalid) -> Delay26/1, Mux4/2
  Delay26/1 -> Mux4/3
  Constant13/1 -> dv_del_sel/1(sim_1)
  dv_del_sel/1(in_sel) -> Mux4/1
  Constant18/1 -> sync_del_sel/1(sim_1)
  sync_del_sel/1(in_sel) -> Mux3/1
  Mux5/1 -> Mux10/2
  Delay27/1 -> Mux5/3
  Constant19/1 -> d0_del_sel/1(sim_1)
  d0_del_sel/1(in_sel) -> Mux5/1
  pipeline72/1(q) -> bus_expand4/1(bus_in)
  From8/1 -> Slice3/1
  Slice1/1 -> pipeline71/1(d)
  From33/1 -> Slice1/1
  pipeline71/1(q) -> snap_adc1/13(extra_timestamp)
  From35/1 -> pipeline72/1(d)
  bus_expand4/4(out5) -> snap_adc1/4(in_p0_d3)
  bus_expand4/5(out4) -> snap_adc1/5(in_p0_d4)
  bus_expand4/1(msb_out8) -> snap_adc1/1(in_p0_d0)
  bus_expand4/2(out7) -> snap_adc1/2(in_p0_d1)
  bus_expand4/3(out6) -> snap_adc1/3(in_p0_d2)
  bus_expand4/6(out3) -> snap_adc1/6(in_p0_d5)
  bus_expand4/7(out2) -> snap_adc1/7(in_p0_d6)
  bus_expand4/8(lsb_out1) -> snap_adc1/8(in_p0_d7)
  pipeline67/1(q) -> snap_adc1/11(we)
  pipeline77/1(q) -> pipeline76/1(d)
  pipeline79/1(q) -> snap_adc1/12(trig), pipeline75/1(d)
  Slice3/1 -> pipeline67/1(d), pipeline77/1(d)
  From34/1 -> pipeline79/1(d)
  pipeline75/1(q) -> snap_adc1/9(in_sync)
  pipeline76/1(q) -> snap_adc1/10(in_dv)
  Mux6/1 -> [osc_cos_ddc0]
  pipeline13/1(q) -> munge/1(din), Mux6/2
  munge/1(dout) -> Mux6/3
  Constant28/1 -> osc_munge/1(sim_1)
  osc_munge/1(in_sel) -> Mux6/1
  impulse_gen0/2(dout) -> Mux11/2, munge1/1(din)
  munge1/1(dout) -> Mux11/3
  Constant31/1 -> adc_munge/1(sim_1)
  adc_munge/1(in_sel) -> Mux11/1
  Mux11/1 -> Delay27/1, Mux5/2
  [ct_d256] -> bus_expand8/1(bus_in)
  [ct_pktfreq] -> pipeline80/1(d)
  [ct_pkt_x_index] -> pipeline84/1(d)
  [ct_freq_sel_dv] -> pipeline87/1(d)
  [ct_pktstart] -> pipeline82/1(d)
  [ct_pkt_x_offset] -> pipeline85/1(d)
  [ct_error] -> pipeline88/1(d)
  [ct_sync] -> pipeline86/1(d)
  pipeline80/1(q) -> snap_ct/1(in_pkt_freq)
  pipeline82/1(q) -> snap_ct/2(in_pkt_start)
  pipeline84/1(q) -> snap_ct/3(in_x_idx)
  pipeline85/1(q) -> snap_ct/4(in_x_offset)
  pipeline86/1(q) -> snap_ct/5(in_sync)
  pipeline87/1(q) -> snap_ct/6(in_dv), snap_ct/8(we)
  pipeline88/1(q) -> snap_ct/7(in_err)
  pipeline89/1(q) -> snap_ctd0/16(in_i7)
  pipeline90/1(q) -> snap_ctd0/15(in_r7)
  pipeline91/1(q) -> snap_ctd0/14(in_i6)
  pipeline92/1(q) -> snap_ctd0/13(in_r6)
  pipeline93/1(q) -> snap_ctd0/17(we)
  pipeline97/1(q) -> snap_ctd0/9(in_r4)
  pipeline94/1(q) -> snap_ctd0/12(in_i5)
  pipeline96/1(q) -> snap_ctd0/10(in_i4)
  pipeline95/1(q) -> snap_ctd0/11(in_r5)
  pipeline155/1(q) -> snap_ctd0/8(in_i3)
  pipeline158/1(q) -> snap_ctd0/6(in_i2)
  pipeline156/1(q) -> snap_ctd0/7(in_r3)
  pipeline159/1(q) -> snap_ctd0/5(in_r2)
  pipeline165/1(q) -> snap_ctd0/2(in_i0)
  pipeline168/1(q) -> snap_ctd0/1(in_r0)
  pipeline160/1(q) -> snap_ctd0/4(in_i1)
  pipeline163/1(q) -> snap_ctd0/3(in_r1)
  bus_expand8/1(msb_out16) -> [p0f0]
  bus_expand8/2(out15) -> [p1f0]
  bus_expand8/3(out14) -> [p0f1]
  bus_expand8/5(out12) -> [p0f2]
  bus_expand8/7(out10) -> [p0f3]
  bus_expand8/9(out8) -> [p0f4]
  bus_expand8/11(out6) -> [p0f5]
  bus_expand8/13(out4) -> [p0f6]
  bus_expand8/15(out2) -> [p0f7]
  bus_expand8/4(out13) -> [p1f1]
  bus_expand8/6(out11) -> [p1f2]
  bus_expand8/8(out9) -> [p1f3]
  bus_expand8/10(out7) -> [p1f4]
  bus_expand8/12(out5) -> [p1f5]
  bus_expand8/14(out3) -> [p1f6]
  bus_expand8/16(lsb_out1) -> [p1f7]
  bus_expand5/1(msb_out2) -> pipeline168/1(d)
  bus_expand5/2(lsb_out1) -> pipeline165/1(d)
  bus_expand10/1(msb_out2) -> pipeline163/1(d)
  bus_expand10/2(lsb_out1) -> pipeline160/1(d)
  bus_expand11/1(msb_out2) -> pipeline159/1(d)
  bus_expand11/2(lsb_out1) -> pipeline158/1(d)
  bus_expand15/1(msb_out2) -> pipeline156/1(d)
  bus_expand15/2(lsb_out1) -> pipeline155/1(d)
  bus_expand19/1(msb_out2) -> pipeline97/1(d)
  bus_expand19/2(lsb_out1) -> pipeline96/1(d)
  bus_expand20/1(msb_out2) -> pipeline95/1(d)
  bus_expand20/2(lsb_out1) -> pipeline94/1(d)
  bus_expand21/1(msb_out2) -> pipeline92/1(d)
  bus_expand21/2(lsb_out1) -> pipeline91/1(d)
  bus_expand22/1(msb_out2) -> pipeline90/1(d)
  bus_expand22/2(lsb_out1) -> pipeline89/1(d)
  [p0f0] -> Delay29/1
  [p0f1] -> Delay45/1
  [p0f2] -> Delay46/1
  [p0f3] -> Delay49/1
  [p0f4] -> Delay54/1
  [p0f5] -> Delay55/1
  [p0f6] -> Delay56/1
  [p0f7] -> Delay94/1
  pipeline169/1(q) -> [ct_freq_sel]
  Constant34/1 -> ct_freq_sel/1(sim_1)
  [ct_freq_sel] -> Relational1/1
  [ct_pktfreq] -> Relational1/2
  Relational1/1 -> edge_detect11/1(in), Logical13/1
  [ct_pktstart] -> Delay28/1
  Logical13/1 -> [ct_freq_sel_trig], Register7/3, Register7/1
  Delay28/1 -> Logical13/2
  Register7/1 -> [ct_freq_sel_dv]
  [ct_freq_sel_trig] -> snap_ctd0/18(trig)
  [ct_freq_sel_dv] -> pipeline93/1(d)
  Delay29/1 -> Delay30/1
  Delay30/1 -> bus_expand5/1(bus_in)
  Delay32/1 -> bus_expand10/1(bus_in)
  Delay33/1 -> bus_expand11/1(bus_in)
  Delay34/1 -> bus_expand15/1(bus_in)
  Delay42/1 -> bus_expand21/1(bus_in)
  Delay40/1 -> bus_expand19/1(bus_in)
  Delay41/1 -> bus_expand20/1(bus_in)
  Delay44/1 -> bus_expand22/1(bus_in)
  Delay49/1 -> Delay34/1
  Delay54/1 -> Delay40/1
  Delay46/1 -> Delay33/1
  Delay45/1 -> Delay32/1
  Delay55/1 -> Delay41/1
  Delay56/1 -> Delay42/1
  Delay94/1 -> Delay44/1
  pipeline253/1(q) -> snap_ctd1/16(in_i7)
  pipeline254/1(q) -> snap_ctd1/15(in_r7)
  pipeline255/1(q) -> snap_ctd1/14(in_i6)
  pipeline256/1(q) -> snap_ctd1/13(in_r6)
  pipeline257/1(q) -> snap_ctd1/17(we)
  pipeline261/1(q) -> snap_ctd1/9(in_r4)
  pipeline258/1(q) -> snap_ctd1/12(in_i5)
  pipeline260/1(q) -> snap_ctd1/10(in_i4)
  pipeline259/1(q) -> snap_ctd1/11(in_r5)
  pipeline234/1(q) -> snap_ctd1/8(in_i3)
  pipeline247/1(q) -> snap_ctd1/6(in_i2)
  pipeline235/1(q) -> snap_ctd1/7(in_r3)
  pipeline248/1(q) -> snap_ctd1/5(in_r2)
  pipeline251/1(q) -> snap_ctd1/2(in_i0)
  pipeline252/1(q) -> snap_ctd1/1(in_r0)
  pipeline249/1(q) -> snap_ctd1/4(in_i1)
  pipeline250/1(q) -> snap_ctd1/3(in_r1)
  bus_expand30/1(msb_out2) -> pipeline252/1(d)
  bus_expand30/2(lsb_out1) -> pipeline251/1(d)
  bus_expand23/1(msb_out2) -> pipeline250/1(d)
  bus_expand23/2(lsb_out1) -> pipeline249/1(d)
  bus_expand24/1(msb_out2) -> pipeline248/1(d)
  bus_expand24/2(lsb_out1) -> pipeline247/1(d)
  bus_expand25/1(msb_out2) -> pipeline235/1(d)
  bus_expand25/2(lsb_out1) -> pipeline234/1(d)
  bus_expand26/1(msb_out2) -> pipeline261/1(d)
  bus_expand26/2(lsb_out1) -> pipeline260/1(d)
  bus_expand27/1(msb_out2) -> pipeline259/1(d)
  bus_expand27/2(lsb_out1) -> pipeline258/1(d)
  bus_expand28/1(msb_out2) -> pipeline256/1(d)
  bus_expand28/2(lsb_out1) -> pipeline255/1(d)
  bus_expand29/1(msb_out2) -> pipeline254/1(d)
  bus_expand29/2(lsb_out1) -> pipeline253/1(d)
  [ct_freq_sel_trig] -> snap_ctd1/18(trig)
  [ct_freq_sel_dv] -> pipeline257/1(d)
  Delay95/1 -> Delay96/1
  Delay96/1 -> bus_expand30/1(bus_in)
  Delay97/1 -> bus_expand23/1(bus_in)
  Delay98/1 -> bus_expand24/1(bus_in)
  Delay99/1 -> bus_expand25/1(bus_in)
  Delay102/1 -> bus_expand28/1(bus_in)
  Delay100/1 -> bus_expand26/1(bus_in)
  Delay101/1 -> bus_expand27/1(bus_in)
  Delay103/1 -> bus_expand29/1(bus_in)
  Delay106/1 -> Delay99/1
  Delay107/1 -> Delay100/1
  Delay105/1 -> Delay98/1
  Delay104/1 -> Delay97/1
  Delay108/1 -> Delay101/1
  Delay109/1 -> Delay102/1
  Delay110/1 -> Delay103/1
  [p1f0] -> Delay95/1
  [p1f7] -> Delay110/1
  [p1f6] -> Delay109/1
  [p1f5] -> Delay108/1
  [p1f4] -> Delay107/1
  [p1f1] -> Delay104/1
  [p1f3] -> Delay106/1
  [p1f2] -> Delay105/1
  edge_detect11/1(out) -> Logical16/1
  [sys_rst] -> Logical16/2
  Logical16/1 -> Register7/2
  ct_freq_sel/1(in_freq) -> pipeline169/1(d)
  [ct_freq_sel_trig] -> snap_ct/9(trig)
  Gateway Out5/1 -> To Workspace5/1
  [q_d64_0] -> bus_expand31/1(bus_in)
  [q_dv] -> Gateway Out5/1
  Gateway Out6/1 -> To Workspace6/1
  [q_sync] -> Gateway Out6/1
  Gateway Out7/1 -> To Workspace7/1
  bus_expand31/1(msb_out8) -> Gateway Out7/1
  Gateway Out8/1 -> To Workspace8/1
  Gateway Out9/1 -> To Workspace9/1
  bus_expand31/3(out6) -> Gateway Out9/1
  Gateway Out10/1 -> To Workspace10/1
  bus_expand31/5(out4) -> Gateway Out10/1
  Gateway Out11/1 -> To Workspace11/1
  bus_expand31/7(out2) -> Gateway Out11/1
  Gateway Out12/1 -> To Workspace12/1
  bus_expand31/4(out5) -> Gateway Out12/1
  Gateway Out13/1 -> To Workspace13/1
  bus_expand31/6(out3) -> Gateway Out13/1
  Gateway Out14/1 -> To Workspace14/1
  bus_expand31/8(lsb_out1) -> Gateway Out14/1
  bus_expand31/2(out7) -> Gateway Out8/1
  Gateway Out20/1 -> To Workspace20/1
  bus_expand32/1(msb_out8) -> Gateway Out20/1
  Gateway Out21/1 -> To Workspace15/1
  Gateway Out22/1 -> To Workspace16/1
  bus_expand32/3(out6) -> Gateway Out22/1
  Gateway Out15/1 -> To Workspace17/1
  bus_expand32/5(out4) -> Gateway Out15/1
  Gateway Out16/1 -> To Workspace18/1
  bus_expand32/7(out2) -> Gateway Out16/1
  Gateway Out17/1 -> To Workspace19/1
  bus_expand32/4(out5) -> Gateway Out17/1
  Gateway Out18/1 -> To Workspace21/1
  bus_expand32/6(out3) -> Gateway Out18/1
  Gateway Out19/1 -> To Workspace22/1
  bus_expand32/8(lsb_out1) -> Gateway Out19/1
  bus_expand32/2(out7) -> Gateway Out21/1
  [fdfs_d144_0] -> bus_expand32/1(bus_in)
  Gateway Out24/1 -> To Workspace24/1
  [fdfs_dv] -> Gateway Out24/1
  Gateway Out25/1 -> To Workspace25/1
  [fdfs_sync] -> Gateway Out25/1
  bus_constant/1(dout) -> Mux12/3
  eq0/1(data_out) -> Mux12/2
  Mux12/1 -> quant0/4(coeffs)
  coeff_sel/1(in_sel) -> pipeline262/1(d)
  pipeline262/1(q) -> [coeff_sel]
  Constant35/1 -> coeff_sel/1(sim_1)
  [coeff_sel] -> Mux12/1
  bus_expand33/4(out5) -> pipeline269/1(d)
  bus_expand33/5(out4) -> pipeline268/1(d)
  bus_expand33/2(out7) -> pipeline271/1(d)
  bus_expand33/3(out6) -> pipeline270/1(d)
  bus_expand33/6(out3) -> pipeline267/1(d)
  bus_expand33/7(out2) -> pipeline266/1(d)
  bus_expand33/8(lsb_out1) -> pipeline265/1(d)
  bus_expand33/1(msb_out8) -> pipeline272/1(d)
  pipeline273/1(q) -> snap_mix/11(we)
  pipeline263/1(q) -> snap_mix/10(in_dv)
  pipeline264/1(q) -> snap_mix/9(in_sync)
  pipeline265/1(q) -> snap_mix/8(in_p0_d7)
  pipeline266/1(q) -> snap_mix/7(in_p0_d6)
  pipeline267/1(q) -> snap_mix/6(in_p0_d5)
  pipeline268/1(q) -> snap_mix/5(in_p0_d4)
  pipeline269/1(q) -> snap_mix/4(in_p0_d3)
  pipeline270/1(q) -> snap_mix/3(in_p0_d2)
  pipeline271/1(q) -> snap_mix/2(in_p0_d1)
  pipeline272/1(q) -> snap_mix/1(in_p0_d0)
  [mix_80_0] -> bus_expand33/1(bus_in)
  [mix_dvalid] -> pipeline263/1(d), pipeline273/1(d)
  [mix_sync] -> pipeline264/1(d), snap_mix/12(trig)
  pipeline227/1(q) -> snap_ddc_out/5(we)
  pipeline61/1(q) -> snap_ddc_out/4(in_dv)
  pipeline218/1(q) -> snap_ddc_out/3(in_sync)
  [ddc_dvalid] -> pipeline227/1(d), pipeline61/1(d)
  [ddc_sync] -> snap_ddc_out/6(trig), pipeline218/1(d)
  [ddc_pol0] -> pipeline219/1(d)
  pipeline219/1(q) -> snap_ddc_out/1(in_p0)
  pipeline220/1(q) -> snap_ddc_out/2(in_p1)
  [ddc_pol1] -> pipeline220/1(d)
  Gateway Out26/1 -> To Workspace26/1
  Constant36/1 -> Relational2/2
  Logical17/1 -> Counter10/1
  [sys_rst] -> Logical17/1
  Relational5/1 -> Logical17/2, Gateway Out30/1
  Constant55/1 -> Relational5/2
  Gateway Out29/1 -> To Workspace29/1
  Gateway Out30/1 -> To Workspace30/1
  pipeline221/1(q) -> snap_fft1/4(in_cap_now)
  pipeline222/1(q) -> snap_fft1/3(in_cur_ch)
  [current_channel] -> pipeline222/1(d)
  [capture_now] -> pipeline221/1(d)
  Gateway Out27/1 -> To Workspace27/1
  Gateway Out31/1 -> To Workspace31/1
  Gateway Out32/1 -> To Workspace32/1
  Gateway Out33/1 -> To Workspace33/1
  Gateway Out34/1 -> To Workspace34/1
  Gateway Out35/1 -> To Workspace35/1
  Gateway Out36/1 -> To Workspace36/1
  Gateway Out37/1 -> To Workspace37/1
  Gateway Out38/1 -> To Workspace38/1
  Gateway Out39/1 -> To Workspace39/1
